simulink model "sys"  (R2024b)
settings: Solver=ode45  StartTime=0.0  StopTime=3  RelTol=1e-3
values from neighbouring files (symbols this model uses):
  N = Nm+mp*(x2+L*(theta2*cos(theta)-theta1^2*sin(theta)))    [state_equation.m]
  X = nmpc.variable(6,step)    [MPC_controller.m]    (also in MPC_controller_14D.m = nmpc.variable(12, step); mlp.m = Xy(:,1:6)')
  Y = Xy(:,7:8)'    [mlp.m]
undefined symbols (not defined in the file or in its folder): EKF, MPC_controller, Z
libraries: sm_lib x1025, nesl_utility x197, slpidlib x40, simulink x12, dspfdesign x2
(part 1 of 26)
settings Model: Creator=Tan Dachao  LastModifiedBy=Tan Dachao

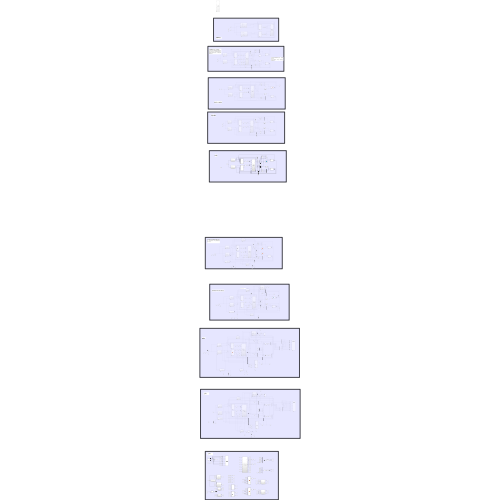
[thumbnail: Simulink's stored preview of the top-level diagram]
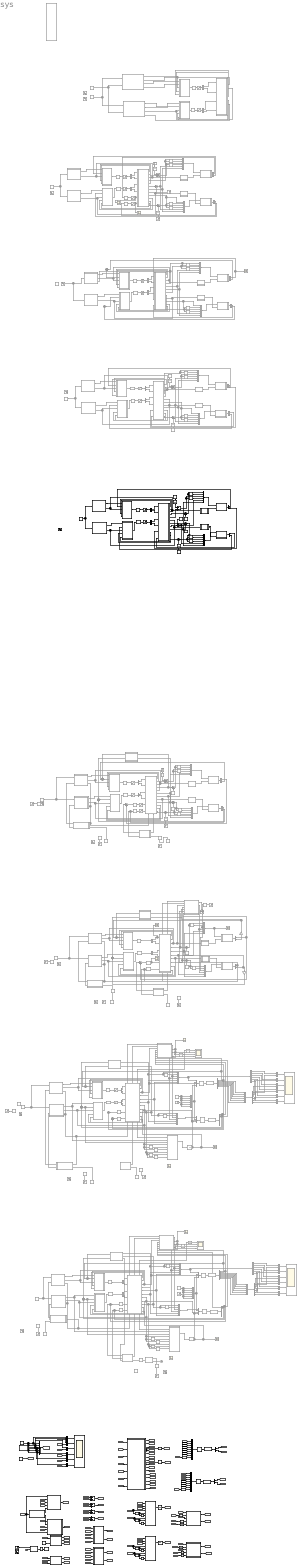
== system sys ==
  note: 反馈，转速一大，始终会有瞬态的角度差
      | 可以考虑推前馈的公式补偿
  note: 这里搭建的simspace是对的，全都按这个参考
      | 这个是后面改的，中间有一些系统没用上懒得改 了
  note: 1234567
  note: 调LQR
  note: 全观测(x未完全实现)
      | 由于轮子在受力瞬间的滑移不能纯靠里程计来计算x，需要融合加速度计才能消除受力瞬间的空转。目前是用Tw限幅来实现
  note: VMC验证
  note: 结构
  note: 调关节参数
  note: 结构
  note: 调关节参数
  note: 6轴
  note: 增加转向环和控制角度差
  note: 增加转向环和控制角度差
      | 这一版是全观测，可以完成3自由度基本控制
  note: MPC
  note: 过坡
  note: 过坡
  Add{,1,3..4} (4 alike) : Sum  Commented=on  Inputs=-+
  Add2 : Sum  Inputs=-+
  Add{5..8} (4 alike) : Sum
  Analog Filter Design : dspfdesign/Analog Filter Design  method=Butterworth  filttype=Lowpass  N=2  Wlo=4  Whi=80  Rp=2  Rs=40
  Clock : Clock  Commented=on
  Clock1 : Clock
  Constant{,3,7..8,16,18..21,23..26,28..29,35} (16 alike) : Constant  Commented=on  Value=0
  Constant1 : Constant  Commented=on  Value=0.13
  Constant10 : Constant  Commented=on  Value=0.18
  Constant11 : Constant  Commented=on  Value=deg2rad(50)
  Constant12 : Constant  Value=deg2rad(0)
  Constant13 : Constant  Value=0.12
  Constant{5..6,14..15,36..37} (6 alike) : Constant  Value=0
  Constant17 : Constant  Commented=on  Value=0.12
  Constant2 : Constant  Commented=on  Value=0.13
  Constant22 : Constant  Commented=on  Value=0.15
  Constant27 : Constant  Commented=on  Value=0.08
  Constant34 : Constant  Commented=on  Value=0.12
  Constant38 : Constant  Value=0.12
  Constant9 : Constant  Commented=on  Value=0.12
  Delay : Delay  DelayLength=1  InputProcessing=Columns as channels (frame based)
  Delay1 : Delay  DelayLength=1  InputProcessing=Columns as channels (frame based)
  Demux{,1..14,19..26,32,34,38..39} (27 alike) : Demux  Commented=on  Outputs=2
  Demux{15..18,43,46} (6 alike) : Demux  Outputs=2
  Demux{31,33,37,42} (4 alike) : Demux  Commented=on  Outputs=8
  Demux{35..36,40..41} (4 alike) : Demux  Commented=on  Outputs=6
  Derivative{,1..12,17..23,28..37} (30 alike) : Derivative  Commented=on
  Derivative{13..16,38..47} (14 alike) : Derivative
  EKF1 : Subsystem
  EKF2 : Subsystem
  EKF_x1 : Subsystem  Commented=on
  EKF_x2 : Subsystem  Commented=on
  EKF_x5 : Subsystem  Commented=on
  Fl1 : Subsystem  Commented=on
      settings: PortSchema="{\"entries\":[{\"content\":{\"sides\":[{\"content\":{\"connectorIds\":[\"In1\"]},\"type\":\"ConnectorPlacement.EquallySpacedRectSide\",\"uuid\":\"1da95429-f45f-47f6-8988-21d04e9d7301\"},{\"content\":{\"connectorIds\":[\"Out1\",\"In2\"],\"side\":\"RIGHT\"},\"type\":\"ConnectorPlacement.EquallySpacedRectSide\",\"uuid\":\"91f268de-d4f8-47bf-a89c-ce608da26a7f\"},{\"content\":{\"side\":\"TOP\"},\"type\":\"ConnectorPlacement.EquallySpacedRectSide\",\"uuid\":\"00e3ca0a-a055-4c98-9a5b-ca3cdd15cacc\"},{\"content\":{\"side\":\"BOTTOM\"},\"type\":\"ConnectorPlacement.EquallySpacedRectSide\",\"uuid\":\"425101d0-2a50-46ee-9f18-05564d8961df\"}]},\"type\":\"ConnectorPlacement.EquallySpacedConnector\",\"uuid\":\"7fddf75c-e649-487d-9520-ce1553949b22\"}],\"packageUris\":[\"http://schema.mathworks.com/mf0/Connector/R2020b\"],\"version\":\"1.0\"}"
  Fl10 : Subsystem  Commented=on
      settings: PortSchema="{\"entries\":[{\"content\":{\"sides\":[{\"content\":{\"connectorIds\":[\"In1\",\"In2\"]},\"type\":\"ConnectorPlacement.EquallySpacedRectSide\",\"uuid\":\"1da95429-f45f-47f6-8988-21d04e9d7301\"},{\"content\":{\"connectorIds\":[\"Out1\",\"In3\",\"In4\"],\"side\":\"RIGHT\"},\"type\":\"ConnectorPlacement.EquallySpacedRectSide\",\"uuid\":\"91f268de-d4f8-47bf-a89c-ce608da26a7f\"},{\"content\":{\"side\":\"TOP\"},\"type\":\"ConnectorPlacement.EquallySpacedRectSide\",\"uuid\":\"00e3ca0a-a055-4c98-9a5b-ca3cdd15cacc\"},{\"content\":{\"side\":\"BOTTOM\"},\"type\":\"ConnectorPlacement.EquallySpacedRectSide\",\"uuid\":\"425101d0-2a50-46ee-9f18-05564d8961df\"}]},\"type\":\"ConnectorPlacement.EquallySpacedConnector\",\"uuid\":\"7fddf75c-e649-487d-9520-ce1553949b22\"}],\"packageUris\":[\"http://schema.mathworks.com/mf0/Connector/R2020b\"],\"version\":\"1.0\"}"
  Fl13 : Subsystem  Commented=on
      settings: PortSchema="{\"entries\":[{\"content\":{\"sides\":[{\"content\":{\"connectorIds\":[\"In1\",\"In2\"]},\"type\":\"ConnectorPlacement.EquallySpacedRectSide\",\"uuid\":\"1da95429-f45f-47f6-8988-21d04e9d7301\"},{\"content\":{\"connectorIds\":[\"Out1\",\"In3\",\"In4\"],\"side\":\"RIGHT\"},\"type\":\"ConnectorPlacement.EquallySpacedRectSide\",\"uuid\":\"91f268de-d4f8-47bf-a89c-ce608da26a7f\"},{\"content\":{\"side\":\"TOP\"},\"type\":\"ConnectorPlacement.EquallySpacedRectSide\",\"uuid\":\"00e3ca0a-a055-4c98-9a5b-ca3cdd15cacc\"},{\"content\":{\"side\":\"BOTTOM\"},\"type\":\"ConnectorPlacement.EquallySpacedRectSide\",\"uuid\":\"425101d0-2a50-46ee-9f18-05564d8961df\"}]},\"type\":\"ConnectorPlacement.EquallySpacedConnector\",\"uuid\":\"7fddf75c-e649-487d-9520-ce1553949b22\"}],\"packageUris\":[\"http://schema.mathworks.com/mf0/Connector/R2020b\"],\"version\":\"1.0\"}"
  Fl14 : Subsystem  Commented=on
      settings: PortSchema="{\"entries\":[{\"content\":{\"sides\":[{\"content\":{\"connectorIds\":[\"In1\",\"In2\"]},\"type\":\"ConnectorPlacement.EquallySpacedRectSide\",\"uuid\":\"1da95429-f45f-47f6-8988-21d04e9d7301\"},{\"content\":{\"connectorIds\":[\"Out1\",\"In3\",\"In4\"],\"side\":\"RIGHT\"},\"type\":\"ConnectorPlacement.EquallySpacedRectSide\",\"uuid\":\"91f268de-d4f8-47bf-a89c-ce608da26a7f\"},{\"content\":{\"side\":\"TOP\"},\"type\":\"ConnectorPlacement.EquallySpacedRectSide\",\"uuid\":\"00e3ca0a-a055-4c98-9a5b-ca3cdd15cacc\"},{\"content\":{\"side\":\"BOTTOM\"},\"type\":\"ConnectorPlacement.EquallySpacedRectSide\",\"uuid\":\"425101d0-2a50-46ee-9f18-05564d8961df\"}]},\"type\":\"ConnectorPlacement.EquallySpacedConnector\",\"uuid\":\"7fddf75c-e649-487d-9520-ce1553949b22\"}],\"packageUris\":[\"http://schema.mathworks.com/mf0/Connector/R2020b\"],\"version\":\"1.0\"}"
  Fl15 : Subsystem  Commented=on
      settings: PortSchema="{\"entries\":[{\"content\":{\"sides\":[{\"content\":{\"connectorIds\":[\"In1\",\"In2\"]},\"type\":\"ConnectorPlacement.EquallySpacedRectSide\",\"uuid\":\"1da95429-f45f-47f6-8988-21d04e9d7301\"},{\"content\":{\"connectorIds\":[\"Out1\",\"In3\",\"In4\",\"In5\"],\"side\":\"RIGHT\"},\"type\":\"ConnectorPlacement.EquallySpacedRectSide\",\"uuid\":\"91f268de-d4f8-47bf-a89c-ce608da26a7f\"},{\"content\":{\"side\":\"TOP\"},\"type\":\"ConnectorPlacement.EquallySpacedRectSide\",\"uuid\":\"00e3ca0a-a055-4c98-9a5b-ca3cdd15cacc\"},{\"content\":{\"side\":\"BOTTOM\"},\"type\":\"ConnectorPlacement.EquallySpacedRectSide\",\"uuid\":\"425101d0-2a50-46ee-9f18-05564d8961df\"}]},\"type\":\"ConnectorPlacement.EquallySpacedConnector\",\"uuid\":\"7fddf75c-e649-487d-9520-ce1553949b22\"}],\"packageUris\":[\"http://schema.mathworks.com/mf0/Connector/R2020b\"],\"version\":\"1.0\"}"
  Fl16 : Subsystem  Commented=on
      settings: PortSchema="{\"entries\":[{\"content\":{\"sides\":[{\"content\":{\"connectorIds\":[\"In1\",\"In2\"]},\"type\":\"ConnectorPlacement.EquallySpacedRectSide\",\"uuid\":\"1da95429-f45f-47f6-8988-21d04e9d7301\"},{\"content\":{\"connectorIds\":[\"Out1\",\"In3\",\"In4\"],\"side\":\"RIGHT\"},\"type\":\"ConnectorPlacement.EquallySpacedRectSide\",\"uuid\":\"91f268de-d4f8-47bf-a89c-ce608da26a7f\"},{\"content\":{\"side\":\"TOP\"},\"type\":\"ConnectorPlacement.EquallySpacedRectSide\",\"uuid\":\"00e3ca0a-a055-4c98-9a5b-ca3cdd15cacc\"},{\"content\":{\"side\":\"BOTTOM\"},\"type\":\"ConnectorPlacement.EquallySpacedRectSide\",\"uuid\":\"425101d0-2a50-46ee-9f18-05564d8961df\"}]},\"type\":\"ConnectorPlacement.EquallySpacedConnector\",\"uuid\":\"7fddf75c-e649-487d-9520-ce1553949b22\"}],\"packageUris\":[\"http://schema.mathworks.com/mf0/Connector/R2020b\"],\"version\":\"1.0\"}"
  Fl17 : Subsystem
  Fl18 : Subsystem
  Fl2 : Subsystem  Commented=on
      settings: PortSchema="{\"entries\":[{\"content\":{\"sides\":[{\"content\":{\"connectorIds\":[\"In1\"]},\"type\":\"ConnectorPlacement.EquallySpacedRectSide\",\"uuid\":\"1da95429-f45f-47f6-8988-21d04e9d7301\"},{\"content\":{\"connectorIds\":[\"Out1\",\"In2\"],\"side\":\"RIGHT\"},\"type\":\"ConnectorPlacement.EquallySpacedRectSide\",\"uuid\":\"91f268de-d4f8-47bf-a89c-ce608da26a7f\"},{\"content\":{\"side\":\"TOP\"},\"type\":\"ConnectorPlacement.EquallySpacedRectSide\",\"uuid\":\"00e3ca0a-a055-4c98-9a5b-ca3cdd15cacc\"},{\"content\":{\"side\":\"BOTTOM\"},\"type\":\"ConnectorPlacement.EquallySpacedRectSide\",\"uuid\":\"425101d0-2a50-46ee-9f18-05564d8961df\"}]},\"type\":\"ConnectorPlacement.EquallySpacedConnector\",\"uuid\":\"7fddf75c-e649-487d-9520-ce1553949b22\"}],\"packageUris\":[\"http://schema.mathworks.com/mf0/Connector/R2020b\"],\"version\":\"1.0\"}"
  Fl3 : Subsystem  Commented=on
      settings: PortSchema="{\"entries\":[{\"content\":{\"sides\":[{\"content\":{\"connectorIds\":[\"In1\"]},\"type\":\"ConnectorPlacement.EquallySpacedRectSide\",\"uuid\":\"1da95429-f45f-47f6-8988-21d04e9d7301\"},{\"content\":{\"connectorIds\":[\"Out1\",\"In2\"],\"side\":\"RIGHT\"},\"type\":\"ConnectorPlacement.EquallySpacedRectSide\",\"uuid\":\"91f268de-d4f8-47bf-a89c-ce608da26a7f\"},{\"content\":{\"side\":\"TOP\"},\"type\":\"ConnectorPlacement.EquallySpacedRectSide\",\"uuid\":\"00e3ca0a-a055-4c98-9a5b-ca3cdd15cacc\"},{\"content\":{\"side\":\"BOTTOM\"},\"type\":\"ConnectorPlacement.EquallySpacedRectSide\",\"uuid\":\"425101d0-2a50-46ee-9f18-05564d8961df\"}]},\"type\":\"ConnectorPlacement.EquallySpacedConnector\",\"uuid\":\"7fddf75c-e649-487d-9520-ce1553949b22\"}],\"packageUris\":[\"http://schema.mathworks.com/mf0/Connector/R2020b\"],\"version\":\"1.0\"}"
  Fl4 : Subsystem  Commented=on
      settings: PortSchema="{\"entries\":[{\"content\":{\"sides\":[{\"content\":{\"connectorIds\":[\"In1\"]},\"type\":\"ConnectorPlacement.EquallySpacedRectSide\",\"uuid\":\"1da95429-f45f-47f6-8988-21d04e9d7301\"},{\"content\":{\"connectorIds\":[\"Out1\",\"In2\"],\"side\":\"RIGHT\"},\"type\":\"ConnectorPlacement.EquallySpacedRectSide\",\"uuid\":\"91f268de-d4f8-47bf-a89c-ce608da26a7f\"},{\"content\":{\"side\":\"TOP\"},\"type\":\"ConnectorPlacement.EquallySpacedRectSide\",\"uuid\":\"00e3ca0a-a055-4c98-9a5b-ca3cdd15cacc\"},{\"content\":{\"side\":\"BOTTOM\"},\"type\":\"ConnectorPlacement.EquallySpacedRectSide\",\"uuid\":\"425101d0-2a50-46ee-9f18-05564d8961df\"}]},\"type\":\"ConnectorPlacement.EquallySpacedConnector\",\"uuid\":\"7fddf75c-e649-487d-9520-ce1553949b22\"}],\"packageUris\":[\"http://schema.mathworks.com/mf0/Connector/R2020b\"],\"version\":\"1.0\"}"
  Fl5 : Subsystem
      settings: PortSchema="{\"entries\":[{\"content\":{\"sides\":[{\"content\":{\"connectorIds\":[\"In1\"]},\"type\":\"ConnectorPlacement.EquallySpacedRectSide\",\"uuid\":\"1da95429-f45f-47f6-8988-21d04e9d7301\"},{\"content\":{\"connectorIds\":[\"Out1\",\"In2\"],\"side\":\"RIGHT\"},\"type\":\"ConnectorPlacement.EquallySpacedRectSide\",\"uuid\":\"91f268de-d4f8-47bf-a89c-ce608da26a7f\"},{\"content\":{\"side\":\"TOP\"},\"type\":\"ConnectorPlacement.EquallySpacedRectSide\",\"uuid\":\"00e3ca0a-a055-4c98-9a5b-ca3cdd15cacc\"},{\"content\":{\"side\":\"BOTTOM\"},\"type\":\"ConnectorPlacement.EquallySpacedRectSide\",\"uuid\":\"425101d0-2a50-46ee-9f18-05564d8961df\"}]},\"type\":\"ConnectorPlacement.EquallySpacedConnector\",\"uuid\":\"7fddf75c-e649-487d-9520-ce1553949b22\"}],\"packageUris\":[\"http://schema.mathworks.com/mf0/Connector/R2020b\"],\"version\":\"1.0\"}"
  Fl6 : Subsystem
      settings: PortSchema="{\"entries\":[{\"content\":{\"sides\":[{\"content\":{\"connectorIds\":[\"In1\"]},\"type\":\"ConnectorPlacement.EquallySpacedRectSide\",\"uuid\":\"1da95429-f45f-47f6-8988-21d04e9d7301\"},{\"content\":{\"connectorIds\":[\"Out1\",\"In2\"],\"side\":\"RIGHT\"},\"type\":\"ConnectorPlacement.EquallySpacedRectSide\",\"uuid\":\"91f268de-d4f8-47bf-a89c-ce608da26a7f\"},{\"content\":{\"side\":\"TOP\"},\"type\":\"ConnectorPlacement.EquallySpacedRectSide\",\"uuid\":\"00e3ca0a-a055-4c98-9a5b-ca3cdd15cacc\"},{\"content\":{\"side\":\"BOTTOM\"},\"type\":\"ConnectorPlacement.EquallySpacedRectSide\",\"uuid\":\"425101d0-2a50-46ee-9f18-05564d8961df\"}]},\"type\":\"ConnectorPlacement.EquallySpacedConnector\",\"uuid\":\"7fddf75c-e649-487d-9520-ce1553949b22\"}],\"packageUris\":[\"http://schema.mathworks.com/mf0/Connector/R2020b\"],\"version\":\"1.0\"}"
  Fl7 : Subsystem  Commented=on
      settings: PortSchema="{\"entries\":[{\"content\":{\"sides\":[{\"content\":{\"connectorIds\":[\"In1\",\"In2\"]},\"type\":\"ConnectorPlacement.EquallySpacedRectSide\",\"uuid\":\"1da95429-f45f-47f6-8988-21d04e9d7301\"},{\"content\":{\"connectorIds\":[\"Out1\",\"In3\",\"In4\"],\"side\":\"RIGHT\"},\"type\":\"ConnectorPlacement.EquallySpacedRectSide\",\"uuid\":\"91f268de-d4f8-47bf-a89c-ce608da26a7f\"},{\"content\":{\"side\":\"TOP\"},\"type\":\"ConnectorPlacement.EquallySpacedRectSide\",\"uuid\":\"00e3ca0a-a055-4c98-9a5b-ca3cdd15cacc\"},{\"content\":{\"side\":\"BOTTOM\"},\"type\":\"ConnectorPlacement.EquallySpacedRectSide\",\"uuid\":\"425101d0-2a50-46ee-9f18-05564d8961df\"}]},\"type\":\"ConnectorPlacement.EquallySpacedConnector\",\"uuid\":\"7fddf75c-e649-487d-9520-ce1553949b22\"}],\"packageUris\":[\"http://schema.mathworks.com/mf0/Connector/R2020b\"],\"version\":\"1.0\"}"
  Fl8 : Subsystem  Commented=on
      settings: PortSchema="{\"entries\":[{\"content\":{\"sides\":[{\"content\":{\"connectorIds\":[\"In1\",\"In2\"]},\"type\":\"ConnectorPlacement.EquallySpacedRectSide\",\"uuid\":\"1da95429-f45f-47f6-8988-21d04e9d7301\"},{\"content\":{\"connectorIds\":[\"Out1\",\"In3\",\"In4\"],\"side\":\"RIGHT\"},\"type\":\"ConnectorPlacement.EquallySpacedRectSide\",\"uuid\":\"91f268de-d4f8-47bf-a89c-ce608da26a7f\"},{\"content\":{\"side\":\"TOP\"},\"type\":\"ConnectorPlacement.EquallySpacedRectSide\",\"uuid\":\"00e3ca0a-a055-4c98-9a5b-ca3cdd15cacc\"},{\"content\":{\"side\":\"BOTTOM\"},\"type\":\"ConnectorPlacement.EquallySpacedRectSide\",\"uuid\":\"425101d0-2a50-46ee-9f18-05564d8961df\"}]},\"type\":\"ConnectorPlacement.EquallySpacedConnector\",\"uuid\":\"7fddf75c-e649-487d-9520-ce1553949b22\"}],\"packageUris\":[\"http://schema.mathworks.com/mf0/Connector/R2020b\"],\"version\":\"1.0\"}"
  Fl9 : Subsystem  Commented=on
      settings: PortSchema="{\"entries\":[{\"content\":{\"sides\":[{\"content\":{\"connectorIds\":[\"In1\",\"In2\"]},\"type\":\"ConnectorPlacement.EquallySpacedRectSide\",\"uuid\":\"1da95429-f45f-47f6-8988-21d04e9d7301\"},{\"content\":{\"connectorIds\":[\"Out1\",\"In3\",\"In4\"],\"side\":\"RIGHT\"},\"type\":\"ConnectorPlacement.EquallySpacedRectSide\",\"uuid\":\"91f268de-d4f8-47bf-a89c-ce608da26a7f\"},{\"content\":{\"side\":\"TOP\"},\"type\":\"ConnectorPlacement.EquallySpacedRectSide\",\"uuid\":\"00e3ca0a-a055-4c98-9a5b-ca3cdd15cacc\"},{\"content\":{\"side\":\"BOTTOM\"},\"type\":\"ConnectorPlacement.EquallySpacedRectSide\",\"uuid\":\"425101d0-2a50-46ee-9f18-05564d8961df\"}]},\"type\":\"ConnectorPlacement.EquallySpacedConnector\",\"uuid\":\"7fddf75c-e649-487d-9520-ce1553949b22\"}],\"packageUris\":[\"http://schema.mathworks.com/mf0/Connector/R2020b\"],\"version\":\"1.0\"}"
  Gain1 : Gain  Commented=on  Gain=-1
  Gain2 : Gain  Commented=on  Gain=-1
  Integrator{,1..4,9..13} (10 alike) : Integrator  Commented=on
  Integrator5 : Integrator
  Integrator6 : Integrator
  Interpreted MATLAB Function{,1..3} (4 alike) : MATLABFcn  Commented=on  MATLABFcn=MPC_controller(u(1:6),u(7:12),u(13),u(14))  OutputDimensions=8
  Interpreted MATLAB Function4 : MATLABFcn  MATLABFcn=MPC_controller(u(1:6),u(7:12),u(13),u(14))  OutputDimensions=2
  Interpreted MATLAB Function5 : MATLABFcn  MATLABFcn=MPC_controller(u(1:6),u(7:12),u(13),u(14))  OutputDimensions=2
  MATLAB Function : MATLAB Function  Commented=on  TreatAsAtomicUnit=on
  MATLAB Function1 : MATLAB Function  Commented=on  TreatAsAtomicUnit=on
  MATLAB Function10 : MATLAB Function  Commented=on  TreatAsAtomicUnit=on
  MATLAB Function11 : MATLAB Function  Commented=on  TreatAsAtomicUnit=on
  MATLAB Function12 : MATLAB Function  Commented=on  TreatAsAtomicUnit=on
  MATLAB Function13 : MATLAB Function  Commented=on  TreatAsAtomicUnit=on
  MATLAB Function14 : MATLAB Function  Commented=on  TreatAsAtomicUnit=on
  MATLAB Function15 : MATLAB Function  Commented=on  TreatAsAtomicUnit=on
  MATLAB Function16 : MATLAB Function  Commented=on  TreatAsAtomicUnit=on
  MATLAB Function17 : MATLAB Function  Commented=on  TreatAsAtomicUnit=on
  MATLAB Function18 : MATLAB Function  Commented=on  TreatAsAtomicUnit=on
  MATLAB Function19 : MATLAB Function  Commented=on  TreatAsAtomicUnit=on
  MATLAB Function2 : MATLAB Function  Commented=on  TreatAsAtomicUnit=on
  MATLAB Function20 : MATLAB Function  TreatAsAtomicUnit=on
  MATLAB Function21 : MATLAB Function  TreatAsAtomicUnit=on
  MATLAB Function22 : MATLAB Function  TreatAsAtomicUnit=on
  MATLAB Function23 : MATLAB Function  TreatAsAtomicUnit=on
  MATLAB Function24 : MATLAB Function  TreatAsAtomicUnit=on
  MATLAB Function25 : MATLAB Function  TreatAsAtomicUnit=on
  MATLAB Function26 : MATLAB Function  Commented=on  TreatAsAtomicUnit=on
  MATLAB Function27 : MATLAB Function  Commented=on  TreatAsAtomicUnit=on
  MATLAB Function28 : MATLAB Function  Commented=on  TreatAsAtomicUnit=on
  MATLAB Function29 : MATLAB Function  Commented=on  TreatAsAtomicUnit=on
  MATLAB Function3 : MATLAB Function  Commented=on  TreatAsAtomicUnit=on
  MATLAB Function30 : MATLAB Function  Commented=on  TreatAsAtomicUnit=on
  MATLAB Function31 : MATLAB Function  Commented=on  TreatAsAtomicUnit=on
  MATLAB Function32 : MATLAB Function  Commented=on  TreatAsAtomicUnit=on
  MATLAB Function33 : MATLAB Function  Commented=on  TreatAsAtomicUnit=on
  MATLAB Function34 : MATLAB Function  Commented=on  TreatAsAtomicUnit=on
  MATLAB Function35 : MATLAB Function  Commented=on  TreatAsAtomicUnit=on
  MATLAB Function36 : MATLAB Function  Commented=on  TreatAsAtomicUnit=on
  MATLAB Function37 : MATLAB Function  Commented=on  TreatAsAtomicUnit=on
  MATLAB Function4 : MATLAB Function  Commented=on  TreatAsAtomicUnit=on
  MATLAB Function44 : MATLAB Function  Commented=on  TreatAsAtomicUnit=on
  MATLAB Function45 : MATLAB Function  Commented=on  TreatAsAtomicUnit=on
  MATLAB Function46 : MATLAB Function  Commented=on  TreatAsAtomicUnit=on
  MATLAB Function47 : MATLAB Function  Commented=on  TreatAsAtomicUnit=on
  MATLAB Function48 : MATLAB Function  Commented=on  TreatAsAtomicUnit=on
  MATLAB Function49 : MATLAB Function  Commented=on  TreatAsAtomicUnit=on
  MATLAB Function5 : MATLAB Function  Commented=on  TreatAsAtomicUnit=on
  MATLAB Function50 : MATLAB Function  Commented=on  TreatAsAtomicUnit=on
  MATLAB Function51 : MATLAB Function  TreatAsAtomicUnit=on
  MATLAB Function52 : MATLAB Function  TreatAsAtomicUnit=on
  MATLAB Function53 : MATLAB Function  TreatAsAtomicUnit=on
  MATLAB Function54 : MATLAB Function  TreatAsAtomicUnit=on
  MATLAB Function55 : MATLAB Function  TreatAsAtomicUnit=on
  MATLAB Function6 : MATLAB Function  Commented=on  TreatAsAtomicUnit=on
  MATLAB Function7 : MATLAB Function  Commented=on  TreatAsAtomicUnit=on
  MATLAB Function8 : MATLAB Function  Commented=on  TreatAsAtomicUnit=on
  MATLAB Function9 : MATLAB Function  Commented=on  TreatAsAtomicUnit=on
  Mux{,18,25,29} (4 alike) : Mux  Commented=on
  Mux{1..6,9..12,15..17,26..28,36..37} (18 alike) : Mux  Commented=on  Inputs=6
  Mux{19..24,30..35} (12 alike) : Mux  Commented=on  Inputs=2
  Mux38 : Mux  Inputs=9
  Mux39 : Mux  Inputs=9
  Mux41 : Mux  Inputs=6
  Mux{43..48} (6 alike) : Mux  Inputs=2
  Mux7 : Mux  Inputs=6
  Mux8 : Mux  Inputs=6
  Rate Limiter : RateLimiter  Commented=on  RisingSlewLimit=3  FallingSlewLimit=-3  SampleTimeMode=inherited
  Rate Limiter10 : RateLimiter  Commented=on  RisingSlewLimit=2  FallingSlewLimit=-2  SampleTimeMode=inherited
  Rate Limiter11 : RateLimiter  Commented=on  RisingSlewLimit=5  FallingSlewLimit=-5  SampleTimeMode=inherited
  Rate Limiter12 : RateLimiter  Commented=on  SampleTimeMode=inherited
  Rate Limiter13 : RateLimiter  RisingSlewLimit=15  FallingSlewLimit=-15  SampleTimeMode=inherited
  Rate Limiter2 : RateLimiter  Commented=on  RisingSlewLimit=0.5  FallingSlewLimit=-2.5  SampleTimeMode=inherited
  Rate Limiter22 : RateLimiter  Commented=on  RisingSlewLimit=3  FallingSlewLimit=-3  SampleTimeMode=inherited
  Rate Limiter3 : RateLimiter  Commented=on  RisingSlewLimit=0.1  FallingSlewLimit=-0.1  SampleTimeMode=inherited
  Rate Limiter4 : RateLimiter  Commented=on  RisingSlewLimit=2.5  FallingSlewLimit=-2.5  SampleTimeMode=inherited
  Rate Limiter5 : RateLimiter  Commented=on  RisingSlewLimit=0.1  FallingSlewLimit=-0.1  SampleTimeMode=inherited
  Rate Limiter6 : RateLimiter  Commented=on  RisingSlewLimit=4  FallingSlewLimit=-4  SampleTimeMode=inherited
  Rate Limiter7 : RateLimiter  Commented=on  RisingSlewLimit=2  FallingSlewLimit=-2  SampleTimeMode=inherited
  Rate Limiter8 : RateLimiter  Commented=on  RisingSlewLimit=2.5  FallingSlewLimit=-2.5  SampleTimeMode=inherited
  Rate Limiter9 : RateLimiter  Commented=on  RisingSlewLimit=0.1  FallingSlewLimit=-0.1  SampleTimeMode=inherited
  Rate Transition{1..4} (4 alike) : RateTransition  Commented=on  OutPortSampleTime=0.01
  Rate Transition5 : RateTransition  OutPortSampleTime=0.01
  Rate Transition6 : RateTransition  OutPortSampleTime=0.01
  Repeating Sequence Stair3 : simulink/Sources/Repeating Sequence Stair  Commented=on  OutValues="[0.15 0.07 0.07 0.07 0.07 0.07  0.07 0.07 0.07 0.07 0.07 0.07].'"  tsamp=0.15  OutMin=[]  OutMax=[]  OutDataTypeStr=double  OutputDataTypeScalingMode=double  OutDataType=fixdt(0,8)  ConRadixGroup=Best Precision: Vector-wise  OutScaling=2^-12
  Saturation : Saturate  Commented=on  UpperLimit=0.6  LowerLimit=-0.6
  Saturation{1..6} (6 alike) : Saturate  Commented=on  UpperLimit=0.8  LowerLimit=-0.8
  Saturation{10..11,22..23} (4 alike) : Saturate  Commented=on
  Saturation14 : Saturate  Commented=on  UpperLimit=0.06  LowerLimit=-0.06
  Saturation15 : Saturate  Commented=on  UpperLimit=0.06  LowerLimit=-0.06
  Saturation20 : Saturate  Commented=on  UpperLimit=0.07  LowerLimit=-0.07
  Saturation21 : Saturate  Commented=on  UpperLimit=0.07  LowerLimit=-0.07
  Saturation7 : Saturate  UpperLimit=0.8  LowerLimit=-0.8
  Saturation8 : Saturate  UpperLimit=0.8  LowerLimit=-0.8
  Saturation9 : Saturate  Commented=on  UpperLimit=0.6  LowerLimit=-0.6
  Scope{,1,3..7,10,14..15,23..24} (12 alike) : Scope  Commented=on
  Scope11 : Scope  Commented=on  NumInputPorts=6
  Scope16 : Scope  NumInputPorts=6
  Scope2 : Scope  Commented=on  NumInputPorts=4
  Scope8 : Scope  Commented=on  NumInputPorts=4
  Scope9 : Scope  Commented=on  NumInputPorts=6
  Sine Wave : Sin  Commented=on  Amplitude=rad2deg(40)  Frequency=3  SampleTime=0
  Sine Wave1 : Sin  Commented=on  Amplitude=0.04  Bias=0.12  Frequency=4  Phase=pi/2  SampleTime=0
  Sine Wave10 : Sin  Commented=on  Amplitude=deg2rad(40)  Bias=deg2rad(90)  Frequency=4  SampleTime=0
  Sine Wave{2,4,11,13} (4 alike) : Sin  Commented=on  Amplitude=deg2rad(20)  Frequency=3  SampleTime=0
  Sine Wave12 : Sin  Commented=on  Amplitude=0.05  Bias=0.12  Frequency=4  SampleTime=0
  Sine Wave15 : Sin  Amplitude=deg2rad(60)  Frequency=2  SampleTime=0
  Sine Wave16 : Sin  Commented=on  Amplitude=0.04  Bias=0.12  Frequency=4  Phase=pi/2  SampleTime=0
  Sine Wave20 : Sin  Commented=on  Amplitude=deg2rad(60)  Frequency=2  SampleTime=0
  Sine Wave3 : Sin  Commented=on  Amplitude=0.04  Bias=0.12  Frequency=4  Phase=pi/2  SampleTime=0
  Sine Wave5 : Sin  Commented=on  Amplitude=0.04  Bias=0.12  Frequency=4  SampleTime=0
  Sine Wave6 : Sin  Amplitude=0.04  Bias=0.12  Frequency=4  Phase=pi/2  SampleTime=0
  Sine Wave7 : Sin  Commented=on  Amplitude=0.065  Bias=0.13  Frequency=4  SampleTime=0
  Sine Wave9 : Sin  Commented=on  Amplitude=0.04  Bias=0.12  Frequency=4  SampleTime=0
  Step : Step  Commented=on  Time=0.3  After=-5  SampleTime=0.01
  Step10 : Step  Commented=on  Before=deg2rad(0)  After=deg2rad(20)  SampleTime=0
  Step11 : Step  Commented=on  Time=0.5  Before=0.1  After=0.15  SampleTime=0
  Step12 : Step  Commented=on  After=-3  SampleTime=0
  Step14 : Step  Commented=on  Time=0.5  Before=0.1  After=0.15  SampleTime=0
  Step15 : Step  Commented=on  Time=0.3  After=deg2rad(180)  SampleTime=0
  Step16 : Step  Commented=on  Time=1.5  After=-1  SampleTime=0.01
  Step18 : Step  Time=0.3  After=5  SampleTime=0
  Step19 : Step  Commented=on  Time=0.3  Before=deg2rad(0)  After=deg2rad(20)  SampleTime=0
  Step2 : Step  Commented=on  Time=0.5  Before=deg2rad(0)  After=deg2rad(20)  SampleTime=0
  Step20 : Step  Commented=on  Time=0.8  After=deg2rad(90)  SampleTime=0
  Step3 : Step  Commented=on  Time=0.5  Before=0.1  After=0.15  SampleTime=0
  Step4 : Step  Commented=on  Time=0.5  Before=deg2rad(0)  After=deg2rad(20)  SampleTime=0
  Step5 : Step  Commented=on  Time=0.5  After=deg2rad(180)  SampleTime=0
  Step7 : Step  Commented=on  Time=0.5  After=0  SampleTime=0
  Step8 : Step  Commented=on  After=-0.5  SampleTime=0
  Step9 : Step  Commented=on  After=-0.5  SampleTime=0
  Subsystem : Subsystem  mask{v_ref=-2, sus_time=2}
      mask DialogControl ParameterGroupVar Prompt: 参数
  Subsystem1 : Subsystem  Commented=on
  Subsystem10 : Subsystem
  Subsystem11 : Subsystem  Commented=on
      settings: PortSchema="{\"entries\":[{\"content\":{\"sides\":[{\"content\":{\"connectorIds\":[\"In1\",\"In2\",\"In3\",\"In4\",\"In5\",\"In6\"]},\"type\":\"ConnectorPlacement.EquallySpacedRectSide\",\"uuid\":\"b07c6593-9a1e-4424-b369-b525e8716f00\"},{\"content\":{\"connectorIds\":[\"Out1\",\"Out2\",\"Out3\",\"Out4\",\"Out5\",\"Out6\",\"Out7\",\"Out8\",\"Out9\",\"Out10\",\"Out11\",\"Out12\"],\"side\":\"RIGHT\"},\"type\":\"ConnectorPlacement.EquallySpacedRectSide\",\"uuid\":\"0709d903-307c-4c19-a02b-ca630625d7db\"},{\"content\":{\"side\":\"TOP\"},\"type\":\"ConnectorPlacement.EquallySpacedRectSide\",\"uuid\":\"bae721a7-cc37-4c15-9cff-411bed113ecb\"},{\"content\":{\"connectorIds\":[\"Out13\",\"Out14\"],\"side\":\"BOTTOM\"},\"type\":\"ConnectorPlacement.EquallySpacedRectSide\",\"uuid\":\"cf85dd5a-81bc-4c60-bccb-68a7a991a35a\"}]},\"type\":\"ConnectorPlacement.EquallySpacedConnector\",\"uuid\":\"9af8dd5a-e43d-46a2-9a7f-0ca12c51dada\"}],\"packageUris\":[\"http://schema.mathworks.com/mf0/Connector/R2020b\"],\"version\":\"1.0\"}"
  Subsystem12 : Subsystem  Commented=on
      settings: PortSchema="{\"entries\":[{\"content\":{\"sides\":[{\"content\":{\"connectorIds\":[\"In1\",\"In2\",\"In3\",\"In4\",\"In5\",\"In6\"]},\"type\":\"ConnectorPlacement.EquallySpacedRectSide\",\"uuid\":\"b07c6593-9a1e-4424-b369-b525e8716f00\"},{\"content\":{\"connectorIds\":[\"Out1\",\"Out2\",\"Out3\",\"Out4\",\"Out5\",\"Out6\",\"Out7\",\"Out8\",\"Out9\",\"Out10\",\"In7\"],\"side\":\"RIGHT\"},\"type\":\"ConnectorPlacement.EquallySpacedRectSide\",\"uuid\":\"0709d903-307c-4c19-a02b-ca630625d7db\"},{\"content\":{\"connectorIds\":[\"Out14\",\"Out15\",\"Out16\"],\"side\":\"TOP\"},\"type\":\"ConnectorPlacement.EquallySpacedRectSide\",\"uuid\":\"bae721a7-cc37-4c15-9cff-411bed113ecb\"},{\"content\":{\"connectorIds\":[\"Out11\",\"Out12\",\"Out13\"],\"side\":\"BOTTOM\"},\"type\":\"ConnectorPlacement.EquallySpacedRectSide\",\"uuid\":\"cf85dd5a-81bc-4c60-bccb-68a7a991a35a\"}]},\"type\":\"ConnectorPlacement.EquallySpacedConnector\",\"uuid\":\"9af8dd5a-e43d-46a2-9a7f-0ca12c51dada\"}],\"packageUris\":[\"http://schema.mathworks.com/mf0/Connector/R2020b\"],\"version\":\"1.0\"}"
  Subsystem13 : Subsystem  Commented=on
      settings: PortSchema="{\"entries\":[{\"content\":{\"sides\":[{\"content\":{\"connectorIds\":[\"In1\",\"In2\",\"In3\",\"In4\",\"In5\",\"In6\"]},\"type\":\"ConnectorPlacement.EquallySpacedRectSide\",\"uuid\":\"b07c6593-9a1e-4424-b369-b525e8716f00\"},{\"content\":{\"connectorIds\":[\"Out1\",\"Out2\",\"Out3\",\"Out4\",\"Out5\",\"Out6\",\"Out7\",\"Out8\",\"Out9\",\"Out10\",\"In7\"],\"side\":\"RIGHT\"},\"type\":\"ConnectorPlacement.EquallySpacedRectSide\",\"uuid\":\"0709d903-307c-4c19-a02b-ca630625d7db\"},{\"content\":{\"connectorIds\":[\"Out14\",\"Out15\",\"Out16\"],\"side\":\"TOP\"},\"type\":\"ConnectorPlacement.EquallySpacedRectSide\",\"uuid\":\"bae721a7-cc37-4c15-9cff-411bed113ecb\"},{\"content\":{\"connectorIds\":[\"Out11\",\"Out12\",\"Out13\"],\"side\":\"BOTTOM\"},\"type\":\"ConnectorPlacement.EquallySpacedRectSide\",\"uuid\":\"cf85dd5a-81bc-4c60-bccb-68a7a991a35a\"}]},\"type\":\"ConnectorPlacement.EquallySpacedConnector\",\"uuid\":\"9af8dd5a-e43d-46a2-9a7f-0ca12c51dada\"}],\"packageUris\":[\"http://schema.mathworks.com/mf0/Connector/R2020b\"],\"version\":\"1.0\"}"
  Subsystem14 : Subsystem  Commented=on
      settings: PortSchema="{\"entries\":[{\"content\":{\"sides\":[{\"content\":{\"connectorIds\":[\"In1\",\"In2\",\"In3\",\"In4\",\"In5\",\"In6\"]},\"type\":\"ConnectorPlacement.EquallySpacedRectSide\",\"uuid\":\"b07c6593-9a1e-4424-b369-b525e8716f00\"},{\"content\":{\"connectorIds\":[\"Out1\",\"Out2\",\"Out3\",\"Out4\",\"Out5\",\"Out6\",\"Out7\",\"Out8\",\"Out9\",\"Out10\"],\"side\":\"RIGHT\"},\"type\":\"ConnectorPlacement.EquallySpacedRectSide\",\"uuid\":\"0709d903-307c-4c19-a02b-ca630625d7db\"},{\"content\":{\"connectorIds\":[\"Out13\",\"Out14\"],\"side\":\"TOP\"},\"type\":\"ConnectorPlacement.EquallySpacedRectSide\",\"uuid\":\"bae721a7-cc37-4c15-9cff-411bed113ecb\"},{\"content\":{\"connectorIds\":[\"Out11\",\"Out12\"],\"side\":\"BOTTOM\"},\"type\":\"ConnectorPlacement.EquallySpacedRectSide\",\"uuid\":\"cf85dd5a-81bc-4c60-bccb-68a7a991a35a\"}]},\"type\":\"ConnectorPlacement.EquallySpacedConnector\",\"uuid\":\"9af8dd5a-e43d-46a2-9a7f-0ca12c51dada\"}],\"packageUris\":[\"http://schema.mathworks.com/mf0/Connector/R2020b\"],\"version\":\"1.0\"}"
  Subsystem15 : Subsystem
  Subsystem2 : Subsystem  Commented=on
  Subsystem3 : Subsystem  Commented=on
  Subsystem4 : Subsystem  Commented=on
      settings: PortSchema="{\"entries\":[{\"content\":{\"sides\":[{\"content\":{\"connectorIds\":[\"In1\",\"In2\"]},\"type\":\"ConnectorPlacement.EquallySpacedRectSide\",\"uuid\":\"1da95429-f45f-47f6-8988-21d04e9d7301\"},{\"content\":{\"connectorIds\":[\"Out1\",\"Out2\",\"In3\",\"In4\"],\"side\":\"RIGHT\"},\"type\":\"ConnectorPlacement.EquallySpacedRectSide\",\"uuid\":\"91f268de-d4f8-47bf-a89c-ce608da26a7f\"},{\"content\":{\"side\":\"TOP\"},\"type\":\"ConnectorPlacement.EquallySpacedRectSide\",\"uuid\":\"00e3ca0a-a055-4c98-9a5b-ca3cdd15cacc\"},{\"content\":{\"side\":\"BOTTOM\"},\"type\":\"ConnectorPlacement.EquallySpacedRectSide\",\"uuid\":\"425101d0-2a50-46ee-9f18-05564d8961df\"}]},\"type\":\"ConnectorPlacement.EquallySpacedConnector\",\"uuid\":\"7fddf75c-e649-487d-9520-ce1553949b22\"}],\"packageUris\":[\"http://schema.mathworks.com/mf0/Connector/R2020b\"],\"version\":\"1.0\"}"